simulink library "lib_all"  (Simulink 7.6)
settings: Solver=ode45  SolverMode=SingleTasking  StartTime=0.0  StopTime=10.0  RelTol=1e-3
values from neighbouring files (symbols this model uses):
  map_lib_03 = [6.8679 7.0159 7.4571 8.1849 9.1892 10.456 11.97 13.71 15.655 17.781 20.063 22.474 ... (803 elements, 73x11)]    [scr_simdata.mat]    (also in scr_simdata_all.mat)
  map_lib_06 = [2.5784 2.6727 2.9588 3.4453 4.1444 5.068 6.226 7.6242 9.2634 11.139 13.24 15.552 ... (803 elements, 73x11)]    [scr_simdata.mat]    (also in scr_simdata_all.mat)
  map_lib_09 = [0.0025784 0.0026727 0.0029588 0.0034453 0.0041444 0.005068 0.006226 0.0076242 0.0092634 0.011139 0.01324 0.015552 ... (803 elements, 73x11)]    [scr_simdata.mat]    (also in scr_simdata_all.mat)
  map_lib_10 = [2.74 2.75 2.76 2.77 2.78 2.79 2.8 2.81 2.82 2.83 2.84 2.85 ... (81 elements, 1x81)]    [scr_simdata.mat]    (also in scr_simdata_all.mat)
  map_lib_11 = [1.1983 1.2027 1.207 1.2113 1.2156 1.2198 1.2241 1.2283 1.2326 1.2368 1.241 1.2451 ... (81 elements, 1x81)]    [scr_simdata.mat]    (also in scr_simdata_all.mat)
  map_lib_12 = [2.74 2.75 2.76 2.77 2.78 2.79 2.8 2.81 2.82 2.83 2.84 2.85 ... (81 elements, 1x81)]    [scr_simdata.mat]    (also in scr_simdata_all.mat)
  map_lib_13 = [1.74 1.75 1.76 1.77 1.78 1.79 1.8 1.81 1.82 1.83 1.84 1.85 ... (81 elements, 1x81)]    [scr_simdata.mat]    (also in scr_simdata_all.mat)
  map_lib_16 = [0.00048828125 1.6159 1.8962 2.0821 2.2251 2.3426 2.4433 2.5318 2.611 2.683 2.749 2.8101 ... (1419 elements, 129x11)]    [scr_simdata.mat]    (also in scr_simdata_all.mat)
  map_mr2_01 = [0 800 1600 2400 3200 4000]    [scr_simdata.mat]    (also in scr_simdata_all.mat)
  map_mr2_02 = [1.32 1.32 1.33 1.34 1.36 1.38]    [scr_simdata.mat]    (also in scr_simdata_all.mat)
  map_mr2_03 = [0 800 1600 2400 3200 4000]    [scr_simdata.mat]    (also in scr_simdata_all.mat)
  map_mr2_04 = [1.32 1.32 1.33 1.34 1.36 1.38]    [scr_simdata.mat]    (also in scr_simdata_all.mat)
  map_mr2_05 = [0 800 1600 2400 3200 4000]    [scr_simdata.mat]    (also in scr_simdata_all.mat)
  map_mr2_06 = [1.32 1.32 1.33 1.34 1.36 1.38]    [scr_simdata.mat]    (also in scr_simdata_all.mat)
  Parameter1 = 6    [scr_simdata.mat]    (also in scr_simdata_all.mat)
  Parameter101 = 30    [scr_simdata.mat]    (also in scr_simdata_all.mat)
  Parameter102 = 12    [scr_simdata.mat]    (also in scr_simdata_all.mat)
  Parameter103 = -240    [scr_simdata.mat]    (also in scr_simdata_all.mat)
  Parameter108 = 48    [scr_simdata.mat]    (also in scr_simdata_all.mat)
  Parameter120 = 16    [scr_simdata.mat]    (also in scr_simdata_all.mat)
  Parameter121 = 87    [scr_simdata.mat]    (also in scr_simdata_all.mat)
  Parameter123 = 78    [scr_simdata.mat]    (also in scr_simdata_all.mat)
  Parameter124 = 60    [scr_simdata.mat]    (also in scr_simdata_all.mat)
  Parameter125 = -480    [scr_simdata.mat]    (also in scr_simdata_all.mat)
  Parameter130 = 96    [scr_simdata.mat]    (also in scr_simdata_all.mat)
  Parameter131 = 3    [scr_simdata.mat]    (also in scr_simdata_all.mat)
  Parameter144 = 3    [scr_simdata.mat]    (also in scr_simdata_all.mat)
  Parameter18 = 128    [scr_simdata.mat]    (also in scr_simdata_all.mat)
  Parameter19 = 16    [scr_simdata.mat]    (also in scr_simdata_all.mat)
  Parameter2 = 12    [scr_simdata.mat]    (also in scr_simdata_all.mat)
  Parameter20 = 7    [scr_simdata.mat]    (also in scr_simdata_all.mat)
  Parameter21 = 4    [scr_simdata.mat]    (also in scr_simdata_all.mat)
  Parameter3 = 18    [scr_simdata.mat]    (also in scr_simdata_all.mat)
  Parameter32 = 1    [scr_simdata.mat]    (also in scr_simdata_all.mat)
  Parameter34 = 24    [scr_simdata.mat]    (also in scr_simdata_all.mat)
  Parameter4 = 138    [scr_simdata.mat]    (also in scr_simdata_all.mat)
  Parameter42 = 48    [scr_simdata.mat]    (also in scr_simdata_all.mat)
  Parameter5 = 135    [scr_simdata.mat]    (also in scr_simdata_all.mat)
  Parameter55 = 0    [scr_simdata.mat]    (also in scr_simdata_all.mat)
  Parameter56 = 0    [scr_simdata.mat]    (also in scr_simdata_all.mat)
  Parameter57 = 1    [scr_simdata.mat]    (also in scr_simdata_all.mat)
  Parameter58 = 1    [scr_simdata.mat]    (also in scr_simdata_all.mat)
  Parameter59 = 2    [scr_simdata.mat]    (also in scr_simdata_all.mat)
  Parameter6 = 132    [scr_simdata.mat]    (also in scr_simdata_all.mat)
  Parameter60 = 2    [scr_simdata.mat]    (also in scr_simdata_all.mat)
  Parameter63 = 144    [scr_simdata.mat]    (also in scr_simdata_all.mat)
  Parameter7 = 126    [scr_simdata.mat]    (also in scr_simdata_all.mat)
  Parameter76 = 64    [scr_simdata.mat]    (also in scr_simdata_all.mat)
  Parameter77 = 135    [scr_simdata.mat]    (also in scr_simdata_all.mat)
  Parameter79 = 126    [scr_simdata.mat]    (also in scr_simdata_all.mat)
  Parameter80 = 108    [scr_simdata.mat]    (also in scr_simdata_all.mat)
  Parameter81 = 0    [scr_simdata.mat]    (also in scr_simdata_all.mat)
  Parameter86 = 0    [scr_simdata.mat]    (also in scr_simdata_all.mat)
  Parameter98 = 112    [scr_simdata.mat]    (also in scr_simdata_all.mat)
  Parameter99 = 39    [scr_simdata.mat]    (also in scr_simdata_all.mat)
undefined symbols (not defined in the file or in its folder): cylvolkp_hih_map2h, cylvolkp_map2h, pk1k_map2h
libraries: simulink x2
(part 6 of 36)

== system lib_all/SubSystem1023/SubSystem1189/SubSystem1194 ==
  note: ***編集禁止***
  element : Inport
  Inside_DSR : DataStoreRead  DataStoreName=DSM97
  Selector : Selector  IndexOptions=Index vector (port)  Indices=[1 3]  OutputSizes=1
  Output : Outport
  wires:
  Selector/1 -> Output/1
  element/1 -> Selector/2
  Inside_DSR/1 -> Selector/1

== system lib_all/SubSystem1023/SubSystem1189/SubSystem1195 ==
  note: ***編集禁止***
  element : Inport
  Inside_DSR : DataStoreRead  DataStoreName=DSM9
  Selector : Selector  IndexOptions=Index vector (port)  Indices=[1 3]  OutputSizes=1
  Output : Outport
  wires:
  Selector/1 -> Output/1
  element/1 -> Selector/2
  Inside_DSR/1 -> Selector/1

== system lib_all/SubSystem1196/SubSystem1200/SubSystem1201 ==
  note: ***編集禁止***
  In1 : Inport
  In2 : Inport  Port=2
  RO1 : RelationalOperator  Operator===  InputSameDT=on
  single1 : DataTypeConversion  OutDataTypeStr=single  SaturateOnIntegerOverflow=on  RndMeth=Zero
  single2 : DataTypeConversion  OutDataTypeStr=single  SaturateOnIntegerOverflow=on  RndMeth=Zero
  Out1 : Outport
  wires:
  RO1/1 -> Out1/1
  In2/1 -> single2/1
  In1/1 -> single1/1
  single1/1 -> RO1/1
  single2/1 -> RO1/2

== system lib_all/SubSystem1196/SubSystem1200/SubSystem1202 ==
  note: ***編集禁止***
  In1 : Inport
  In2 : Inport  Port=2
  RO1 : RelationalOperator  Operator===  InputSameDT=on
  single1 : DataTypeConversion  OutDataTypeStr=single  SaturateOnIntegerOverflow=on  RndMeth=Zero
  single2 : DataTypeConversion  OutDataTypeStr=single  SaturateOnIntegerOverflow=on  RndMeth=Zero
  Out1 : Outport
  wires:
  RO1/1 -> Out1/1
  In2/1 -> single2/1
  In1/1 -> single1/1
  single1/1 -> RO1/1
  single2/1 -> RO1/2

== system lib_all/SubSystem1196/SubSystem1200/SubSystem1203 ==
  note: ***編集禁止***
  In1 : Inport
  In2 : Inport  Port=2
  RO1 : RelationalOperator  Operator===  InputSameDT=on
  single1 : DataTypeConversion  OutDataTypeStr=single  SaturateOnIntegerOverflow=on  RndMeth=Zero
  single2 : DataTypeConversion  OutDataTypeStr=single  SaturateOnIntegerOverflow=on  RndMeth=Zero
  Out1 : Outport
  wires:
  RO1/1 -> Out1/1
  In2/1 -> single2/1
  In1/1 -> single1/1
  single1/1 -> RO1/1
  single2/1 -> RO1/2

== system lib_all/SubSystem1196/SubSystem1200/SubSystem1204 ==
  note: ***編集禁止***
  element : Inport
  Inside_DSR : DataStoreRead  DataStoreName=DSM97
  Selector : Selector  IndexOptions=Index vector (port)  Indices=[1 3]  OutputSizes=1
  Output : Outport
  wires:
  Selector/1 -> Output/1
  element/1 -> Selector/2
  Inside_DSR/1 -> Selector/1

== system lib_all/SubSystem1196/SubSystem1200/SubSystem1205 ==
  note: ***編集禁止***
  element : Inport
  Inside_DSR : DataStoreRead  DataStoreName=DSM9
  Selector : Selector  IndexOptions=Index vector (port)  Indices=[1 3]  OutputSizes=1
  Output : Outport
  wires:
  Selector/1 -> Output/1
  element/1 -> Selector/2
  Inside_DSR/1 -> Selector/1

== system lib_all/SubSystem1196/SubSystem1206/SubSystem1208 ==
  note: Text242
  Block3277 : Inport
  Block3278 : Inport  Port=2
  Block3279 : Inport  Port=3
  Block3280 : Inport  Port=4
  Block3281 : Constant  Value=0  OutDataTypeStr=double  SampleTime=-1
  Block3282 : Constant  OutDataTypeStr=double  SampleTime=-1
  Block3283 : Constant  Value=0  OutDataTypeStr=double  SampleTime=-1
  Block3284 : Gain  Gain=1/5  SaturateOnIntegerOverflow=on  OutDataTypeStr=Inherit: Same as input  ParamDataTypeStr=Inherit: Same as input
      settings: AttributesFormatString=Attribute652
  Block3285 : Product  OutDataTypeStr=double  SaturateOnIntegerOverflow=on  RndMeth=Zero  InputSameDT=on
  Block3286 : Sum  Inputs=-+  OutDataTypeStr=double  RndMeth=Zero  SaturateOnIntegerOverflow=on  InputSameDT=on
  Block3287 : Sum  OutDataTypeStr=double  RndMeth=Zero  SaturateOnIntegerOverflow=on  InputSameDT=on
  Block3288 : Sum  OutDataTypeStr=double  RndMeth=Zero  SaturateOnIntegerOverflow=on  InputSameDT=on
  Block3289 : Switch  Threshold=0.5  RndMeth=Zero  SaturateOnIntegerOverflow=on  InputSameDT=on
  SubSystem1210 : Subsystem
  SubSystem1211 : Subsystem
  SubSystem1217 : Subsystem
  Block3398 : Outport
  wires:
  Block3288/1 -> SubSystem1217/3(Block3319), SubSystem1211/2(Block3291)
  Block3277/1 -> Block3288/1
  Block3284/1 -> Block3287/2
  Block3285/1 -> Block3284/1
  Block3282/1 -> Block3288/2
  Block3281/1 -> Block3289/1
  Block3289/1 -> Block3398/1
  SubSystem1210/1(Out1) -> Block3289/2
  Block3283/1 -> SubSystem1210/2(In2)
  Block3279/1 -> SubSystem1211/3(Block3292)
  Block3287/1 -> SubSystem1210/1(In1), Block3289/3
  SubSystem1211/2(Block3316) -> Block3285/2
  Block3286/1 -> Block3285/1  "SigLabel110"
  SubSystem1217/2(Block3397) -> Block3286/2
  SubSystem1217/1(Block3396) -> Block3286/1, Block3287/1
  Block3280/1 -> SubSystem1217/2(Block3318)
  SubSystem1211/1(Block3315) -> SubSystem1217/1(Block3317)
  Block3278/1 -> SubSystem1211/1(Block3290)

== system lib_all/SubSystem1196/SubSystem1206/SubSystem1261 ==
  note: Text245
  Block3399 : Inport
  Block3400 : Inport  Port=2
  Block3401 : Inport  Port=3
  Block3402 : Inport  Port=4
  SubSystem1263 : Subsystem
  SubSystem1271 : Subsystem
  Block3444 : Outport
  wires:
  Block3401/1 -> SubSystem1263/3(Block3405)
  SubSystem1271/1(Block3443) -> Block3444/1
  Block3402/1 -> SubSystem1271/1(Block3436)
  SubSystem1263/1(Block3435) -> SubSystem1271/2(Block3437)
  Block3399/1 -> SubSystem1263/2(Block3404)
  Block3400/1 -> SubSystem1263/1(Block3403)

== system lib_all/SubSystem1196/SubSystem1273/SubSystem1275 ==
  note: Text254
  Block3452 : Inport
  Block3453 : Inport  Port=2
  Block3454 : Inport  Port=3
  Block3455 : Inport  Port=4
  Block3456 : Constant  Value=0  OutDataTypeStr=double  SampleTime=-1
  Block3457 : Constant  OutDataTypeStr=double  SampleTime=-1
  Block3458 : Constant  Value=0  OutDataTypeStr=double  SampleTime=-1
  Block3459 : Gain  Gain=1/5  SaturateOnIntegerOverflow=on  OutDataTypeStr=Inherit: Same as input  ParamDataTypeStr=Inherit: Same as input
      settings: AttributesFormatString=Attribute685
  Block3460 : Product  OutDataTypeStr=double  SaturateOnIntegerOverflow=on  RndMeth=Zero  InputSameDT=on
  Block3461 : Sum  Inputs=-+  OutDataTypeStr=double  RndMeth=Zero  SaturateOnIntegerOverflow=on  InputSameDT=on
  Block3462 : Sum  OutDataTypeStr=double  RndMeth=Zero  SaturateOnIntegerOverflow=on  InputSameDT=on
  Block3463 : Sum  OutDataTypeStr=double  RndMeth=Zero  SaturateOnIntegerOverflow=on  InputSameDT=on
  Block3464 : Switch  Threshold=0.5  RndMeth=Zero  SaturateOnIntegerOverflow=on  InputSameDT=on
  SubSystem1277 : Subsystem
  SubSystem1278 : Subsystem
  SubSystem1284 : Subsystem
  Block3573 : Outport
  wires:
  Block3463/1 -> SubSystem1284/3(Block3494), SubSystem1278/2(Block3466)
  Block3452/1 -> Block3463/1
  Block3459/1 -> Block3462/2
  Block3460/1 -> Block3459/1
  Block3457/1 -> Block3463/2
  Block3456/1 -> Block3464/1
  Block3464/1 -> Block3573/1
  SubSystem1277/1(Out1) -> Block3464/2
  Block3458/1 -> SubSystem1277/2(In2)
  Block3454/1 -> SubSystem1278/3(Block3467)
  Block3462/1 -> SubSystem1277/1(In1), Block3464/3
  SubSystem1278/2(Block3491) -> Block3460/2
  Block3461/1 -> Block3460/1  "SigLabel116"
  SubSystem1284/2(Block3572) -> Block3461/2
  SubSystem1284/1(Block3571) -> Block3461/1, Block3462/1
  Block3455/1 -> SubSystem1284/2(Block3493)
  SubSystem1278/1(Block3490) -> SubSystem1284/1(Block3492)
  Block3453/1 -> SubSystem1278/1(Block3465)

== system lib_all/SubSystem1196/SubSystem1273/SubSystem1328 ==
  note: Text257
  Block3574 : Inport
  Block3575 : Inport  Port=2
  Block3576 : Inport  Port=3
  Block3577 : Inport  Port=4
  SubSystem1330 : Subsystem
  SubSystem1338 : Subsystem
  Block3619 : Outport
  wires:
  Block3576/1 -> SubSystem1330/3(Block3580)
  SubSystem1338/1(Block3618) -> Block3619/1
  Block3577/1 -> SubSystem1338/1(Block3611)
  SubSystem1330/1(Block3610) -> SubSystem1338/2(Block3612)
  Block3574/1 -> SubSystem1330/2(Block3579)
  Block3575/1 -> SubSystem1330/1(Block3578)

== system lib_all/SubSystem1196/SubSystem1340/SubSystem1342 ==
  note: Text261
  Block3625 : Inport
  Block3626 : Inport  Port=2
  Block3627 : Inport  Port=3
  Block3628 : Constant  Value=0  OutDataTypeStr=double  SampleTime=-1
  Block3629 : Constant  Value=720  OutDataTypeStr=double  SampleTime=-1
  Block3630 : Gain  Gain=5  SaturateOnIntegerOverflow=on  OutDataTypeStr=Inherit: Same as input  ParamDataTypeStr=Inherit: Same as input
      settings: AttributesFormatString=Attribute716
  Block3631 : Sum  Inputs=+-  OutDataTypeStr=double  RndMeth=Zero  SaturateOnIntegerOverflow=on  InputSameDT=on
  SubSystem1343 : Subsystem
      settings: RTWFcnNameOpts=Use subsystem name
  SubSystem1348 : Subsystem
      settings: RTWSystemCode=Inline
  Block3657 : Outport
  wires:
  SubSystem1348/1(Block3656) -> Block3657/1
  Block3628/1 -> SubSystem1348/3(Block3653)
  Block3629/1 -> SubSystem1348/1(Block3651)
  Block3631/1 -> SubSystem1348/2(Block3652)
  Block3627/1 -> Block3631/2
  Block3626/1 -> SubSystem1343/2(Block3633)
  Block3625/1 -> SubSystem1343/1(Block3632)
  SubSystem1343/1(Block3650) -> Block3630/1
  Block3630/1 -> Block3631/1

== system lib_all/SubSystem1196/SubSystem1340/SubSystem1350 ==
  note: Text262
  Block3658 : Inport
  Block3659 : Inport  Port=2
  Block3660 : Constant  Value=360  OutDataTypeStr=double  SampleTime=-1
  Block3661 : Constant  Value=360  OutDataTypeStr=double  SampleTime=-1
  Block3662 : Sum  Inputs=+-  OutDataTypeStr=double  RndMeth=Zero  SaturateOnIntegerOverflow=on  InputSameDT=on
  Block3663 : Switch  Threshold=0.5  RndMeth=Zero  SaturateOnIntegerOverflow=on  InputSameDT=on
  Block3664 : Lookup2D  RowIndex=map_lib_08  ColumnIndex=map_lib_07  Table=map_lib_09  SaturateOnIntegerOverflow=on  InputSameDT=on
      settings: AttributesFormatString=<%<OutputValues>>\nAttribute725
  SubSystem1351 : Subsystem
  Block3665 : Outport
  wires:
  Block3664/1 -> Block3665/1
  Block3660/1 -> Block3662/2
  Block3662/1 -> Block3663/1
  SubSystem1351/1(Out1) -> Block3663/2
  Block3661/1 -> SubSystem1351/2(In2)
  Block3659/1 -> Block3662/1, Block3663/3, SubSystem1351/1(In1)
  Block3658/1 -> Block3664/2
  Block3663/1 -> Block3664/1  "SigLabel120"

== system lib_all/SubSystem1196/SubSystem1352/SubSystem1354 ==
  note: Text264
  Block3671 : Inport
  Block3672 : Inport  Port=2
  Block3673 : Inport  Port=3
  Block3674 : Constant  Value=0  OutDataTypeStr=double  SampleTime=-1
  Block3675 : Constant  Value=720  OutDataTypeStr=double  SampleTime=-1
  Block3676 : Gain  Gain=5  SaturateOnIntegerOverflow=on  OutDataTypeStr=Inherit: Same as input  ParamDataTypeStr=Inherit: Same as input
      settings: AttributesFormatString=Attribute729
  Block3677 : Sum  Inputs=+-  OutDataTypeStr=double  RndMeth=Zero  SaturateOnIntegerOverflow=on  InputSameDT=on
  SubSystem1355 : Subsystem
      settings: RTWFcnNameOpts=Use subsystem name
  SubSystem1360 : Subsystem
      settings: RTWSystemCode=Inline
  Block3703 : Outport
  wires:
  SubSystem1360/1(Block3702) -> Block3703/1
  Block3674/1 -> SubSystem1360/3(Block3699)
  Block3675/1 -> SubSystem1360/1(Block3697)
  Block3677/1 -> SubSystem1360/2(Block3698)
  Block3673/1 -> Block3677/2
  Block3672/1 -> SubSystem1355/2(Block3679)
  Block3671/1 -> SubSystem1355/1(Block3678)
  SubSystem1355/1(Block3696) -> Block3676/1
  Block3676/1 -> Block3677/1

== system lib_all/SubSystem1196/SubSystem1352/SubSystem1362 ==
  note: Text265
  Block3704 : Inport
  Block3705 : Inport  Port=2
  Block3706 : Constant  Value=360  OutDataTypeStr=double  SampleTime=-1
  Block3707 : Constant  Value=360  OutDataTypeStr=double  SampleTime=-1
  Block3708 : Sum  Inputs=+-  OutDataTypeStr=double  RndMeth=Zero  SaturateOnIntegerOverflow=on  InputSameDT=on
  Block3709 : Switch  Threshold=0.5  RndMeth=Zero  SaturateOnIntegerOverflow=on  InputSameDT=on
  Block3710 : Lookup2D  RowIndex=map_lib_08  ColumnIndex=map_lib_07  Table=map_lib_09  SaturateOnIntegerOverflow=on  InputSameDT=on
      settings: AttributesFormatString=<%<OutputValues>>\nAttribute738
  SubSystem1363 : Subsystem
  Block3711 : Outport
  wires:
  Block3710/1 -> Block3711/1
  Block3706/1 -> Block3708/2
  Block3708/1 -> Block3709/1
  SubSystem1363/1(Out1) -> Block3709/2
  Block3707/1 -> SubSystem1363/2(In2)
  Block3705/1 -> Block3708/1, Block3709/3, SubSystem1363/1(In1)
  Block3704/1 -> Block3710/2
  Block3709/1 -> Block3710/1  "SigLabel121"

== system lib_all/SubSystem1364/SubSystem1366/SubSystem1368 ==
  note: ***編集禁止***
  In1 : Inport
  In2 : Inport  Port=2
  LO1 : Logic
  RO1 : RelationalOperator  Operator=<  InputSameDT=on
  RO2 : RelationalOperator  Operator=~=  InputSameDT=on
  single1 : DataTypeConversion  OutDataTypeStr=single  SaturateOnIntegerOverflow=on  RndMeth=Zero
  single2 : DataTypeConversion  OutDataTypeStr=single  SaturateOnIntegerOverflow=on  RndMeth=Zero
  Out1 : Outport
  wires:
  LO1/1 -> Out1/1
  RO2/1 -> LO1/2
  RO1/1 -> LO1/1
  single2/1 -> RO2/2, RO1/2
  single1/1 -> RO2/1, RO1/1
  In1/1 -> single1/1
  In2/1 -> single2/1

== system lib_all/SubSystem1364/SubSystem1366/SubSystem1369 ==
  note: Text270
  note: Text269
  Block3744 : Inport
  Block3745 : Inport  Port=2
  Block3746 : Inport  Port=3
  Block3747 : Constant  Value=720  OutDataTypeStr=double  SampleTime=-1
  Block3748 : Constant  Value=0  OutDataTypeStr=double  SampleTime=-1
  Block3749 : Constant  Value=0  OutDataTypeStr=double  SampleTime=-1
  Block3750 : Constant  Value=5  OutDataTypeStr=double  SampleTime=-1
  Block3751 : DataStoreRead  DataStoreName=DSM50
  Block3752 : DataStoreRead  DataStoreName=DSM35
  Block3753 : Gain  Gain=5  SaturateOnIntegerOverflow=on  OutDataTypeStr=Inherit: Same as input  ParamDataTypeStr=Inherit: Same as input
      settings: AttributesFormatString=Attribute752
  Block3754 : Gain  Gain=5  SaturateOnIntegerOverflow=on  OutDataTypeStr=Inherit: Same as input  ParamDataTypeStr=Inherit: Same as input
      settings: AttributesFormatString=Attribute753
  Block3755 : Gain  Gain=1/5  SaturateOnIntegerOverflow=on  OutDataTypeStr=Inherit: Same as input  ParamDataTypeStr=Inherit: Same as input
      settings: AttributesFormatString=Attribute754
  Block3756 : Product  Inputs=*/  OutDataTypeStr=double  SaturateOnIntegerOverflow=on  RndMeth=Zero  InputSameDT=on
  Block3757 : Rounding
  Block3758 : Rounding
  Block3759 : Sum  Inputs=+-+-  OutDataTypeStr=double  RndMeth=Zero  SaturateOnIntegerOverflow=on  InputSameDT=on
  Block3760 : Sum  Inputs=-+  OutDataTypeStr=double  RndMeth=Zero  SaturateOnIntegerOverflow=on  InputSameDT=on
  SubSystem1370 : Subsystem  TreatAsAtomicUnit=on  mask(***ATC.DIVISION***){bd_version=1.01.A, r0=チェック有り, r1=, r2=, r3=, r4=, r5=, r6=, r7=, r8=, r9=, c0=, c1=, c2=, c3=, c4=, c5=, c6=, c7=, c8=, c9=}
      mask prompts: bd_version "Version"
      mask help: これはAutoCからのブロックです．編集禁止．
      settings: OpenFcn=warndlg('変更禁止!');
      mask Help: web(ui_blockhelpmng(gcb));
  SubSystem1371 : Subsystem  TreatAsAtomicUnit=on  mask(***ATC.DSRVEC***){bd_version=1.01.A, r0=DSM95, r1=-1, r2=, r3=, r4=, r5=, r6=, r7=, r8=, r9=, c0=, c1=, c2=, c3=, c4=, c5=, c6=, c7=, c8=, c9=}
      mask prompts: bd_version "Version"
      mask help: これはAutoCからのブロックです．編集禁止．
      settings: OpenFcn=warndlg('変更禁止!');
      mask Help: web(ui_blockhelpmng(gcb));
      mask Display: disp('ATC.DSRVEC')
  SubSystem1372 : Subsystem
      settings: RTWSystemCode=Inline
  Block3769 : Outport
  Block3770 : Outport  Port=2
  wires:
  Block3752/1 -> SubSystem1370/2(div)
  Block3760/1 -> Block3770/1
  Block3753/1 -> Block3760/1
  Block3757/1 -> Block3753/1
  Block3756/1 -> Block3757/1
  Block3750/1 -> Block3756/2
  Block3745/1 -> SubSystem1371/1(element)
  Block3749/1 -> SubSystem1372/3(Block3763)
  Block3759/1 -> SubSystem1372/2(Block3762)  "SigLabel135"
  Block3747/1 -> SubSystem1372/1(Block3761)
  Block3746/1 -> Block3759/4
  SubSystem1372/1(Block3768) -> Block3756/1, Block3760/2, Block3755/1
  Block3744/1 -> Block3754/1
  SubSystem1370/1(Out) -> Block3759/3  "SigLabel134"
  Block3748/1 -> SubSystem1370/3(init)
  SubSystem1371/1(Output) -> SubSystem1370/1(mult)
  Block3751/1 -> Block3759/2
  Block3754/1 -> Block3759/1
  Block3755/1 -> Block3758/1
  Block3758/1 -> Block3769/1

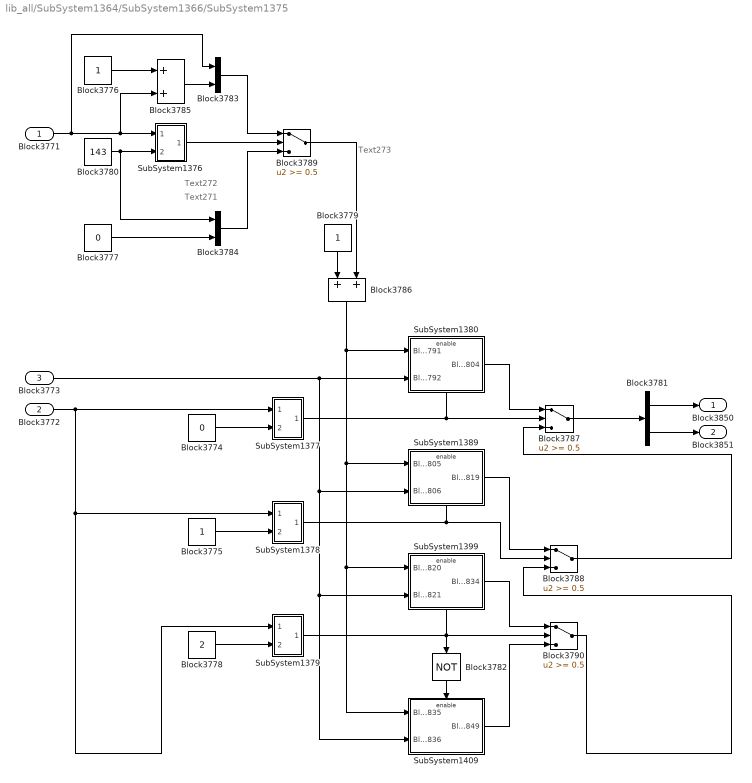
== system lib_all/SubSystem1364/SubSystem1366/SubSystem1375 ==
  note: Text273
  note: Text272
  note: Text271
  Block3771 : Inport
  Block3772 : Inport  Port=2
  Block3773 : Inport  Port=3
  Block3774 : Constant  Value=0  OutDataTypeStr=double  SampleTime=-1
  Block3775 : Constant  OutDataTypeStr=double  SampleTime=-1
  Block3776 : Constant  OutDataTypeStr=double  SampleTime=-1
  Block3777 : Constant  Value=0  OutDataTypeStr=double  SampleTime=-1
  Block3778 : Constant  Value=2  OutDataTypeStr=double  SampleTime=-1
  Block3779 : Constant  OutDataTypeStr=double  SampleTime=-1
  Block3780 : Constant  Value=143  OutDataTypeStr=double  SampleTime=-1
  Block3781 : Demux  Outputs=2
  Block3782 : Logic  Operator=NOT
  Block3783 : Mux  Inputs=2
  Block3784 : Mux  Inputs=2
  Block3785 : Sum  OutDataTypeStr=double  RndMeth=Zero  SaturateOnIntegerOverflow=on  InputSameDT=on
  Block3786 : Sum  OutDataTypeStr=double  RndMeth=Zero  SaturateOnIntegerOverflow=on  InputSameDT=on
  Block{3787..3790} (4 alike) : Switch  Threshold=0.5  RndMeth=Zero  SaturateOnIntegerOverflow=on  InputSameDT=on
  SubSystem1376 : Subsystem
  SubSystem1377 : Subsystem
  SubSystem1378 : Subsystem
  SubSystem1379 : Subsystem
  SubSystem1380 : Subsystem  TreatAsAtomicUnit=on
  SubSystem1389 : Subsystem  TreatAsAtomicUnit=on
  SubSystem1399 : Subsystem  TreatAsAtomicUnit=on
  SubSystem1409 : Subsystem  TreatAsAtomicUnit=on
  Block3850 : Outport
  Block3851 : Outport  Port=2
  wires:
  Block3773/1 -> SubSystem1409/2(Block3836), SubSystem1399/2(Block3821), SubSystem1389/2(Block3806), SubSystem1380/2(Block3792)
  Block3790/1 -> Block3788/3
  SubSystem1409/1(Block3849) -> Block3790/3
  SubSystem1399/1(Block3834) -> Block3790/1
  Block3782/1 -> SubSystem1409/Enable
  SubSystem1379/1(Out1) -> Block3790/2, SubSystem1399/Enable, Block3782/1
  Block3778/1 -> SubSystem1379/2(In2)
  Block3783/1 -> Block3789/1
  Block3785/1 -> Block3783/2
  Block3784/1 -> Block3789/3
  Block3777/1 -> Block3784/2
  Block3776/1 -> Block3785/1
  SubSystem1376/1(Out1) -> Block3789/2
  Block3787/1 -> Block3781/1
  Block3781/2 -> Block3851/1
  Block3781/1 -> Block3850/1
  Block3786/1 -> SubSystem1409/1(Block3835), SubSystem1399/1(Block3820), SubSystem1389/1(Block3805), SubSystem1380/1(Block3791)
  Block3788/1 -> Block3787/3
  SubSystem1389/1(Block3819) -> Block3788/1
  SubSystem1378/1(Out1) -> SubSystem1389/Enable, Block3788/2
  Block3775/1 -> SubSystem1378/2(In2)
  SubSystem1380/1(Block3804) -> Block3787/1
  SubSystem1377/1(Out1) -> SubSystem1380/Enable, Block3787/2
  Block3774/1 -> SubSystem1377/2(In2)
  Block3772/1 -> SubSystem1379/1(In1), SubSystem1378/1(In1), SubSystem1377/1(In1)
  Block3780/1 -> Block3784/1, SubSystem1376/2(In2)
  Block3771/1 -> Block3783/1, Block3785/2, SubSystem1376/1(In1)
  Block3779/1 -> Block3786/1
  Block3789/1 -> Block3786/2

== system lib_all/SubSystem1364/SubSystem1419/SubSystem1421 ==
  note: ***編集禁止***
  In1 : Inport
  In2 : Inport  Port=2
  LO1 : Logic
  RO1 : RelationalOperator  Operator=<  InputSameDT=on
  RO2 : RelationalOperator  Operator=~=  InputSameDT=on
  single1 : DataTypeConversion  OutDataTypeStr=single  SaturateOnIntegerOverflow=on  RndMeth=Zero
  single2 : DataTypeConversion  OutDataTypeStr=single  SaturateOnIntegerOverflow=on  RndMeth=Zero
  Out1 : Outport
  wires:
  LO1/1 -> Out1/1
  RO2/1 -> LO1/2
  RO1/1 -> LO1/1
  single2/1 -> RO2/2, RO1/2
  single1/1 -> RO2/1, RO1/1
  In1/1 -> single1/1
  In2/1 -> single2/1

== system lib_all/SubSystem1364/SubSystem1419/SubSystem1422 ==
  note: Text276
  note: Text275
  Block3866 : Inport
  Block3867 : Inport  Port=2
  Block3868 : Inport  Port=3
  Block3869 : Constant  Value=720  OutDataTypeStr=double  SampleTime=-1
  Block3870 : Constant  Value=0  OutDataTypeStr=double  SampleTime=-1
  Block3871 : Constant  Value=0  OutDataTypeStr=double  SampleTime=-1
  Block3872 : Constant  Value=5  OutDataTypeStr=double  SampleTime=-1
  Block3873 : DataStoreRead  DataStoreName=DSM50
  Block3874 : DataStoreRead  DataStoreName=DSM35
  Block3875 : Gain  Gain=5  SaturateOnIntegerOverflow=on  OutDataTypeStr=Inherit: Same as input  ParamDataTypeStr=Inherit: Same as input
      settings: AttributesFormatString=Attribute771
  Block3876 : Gain  Gain=5  SaturateOnIntegerOverflow=on  OutDataTypeStr=Inherit: Same as input  ParamDataTypeStr=Inherit: Same as input
      settings: AttributesFormatString=Attribute772
  Block3877 : Gain  Gain=1/5  SaturateOnIntegerOverflow=on  OutDataTypeStr=Inherit: Same as input  ParamDataTypeStr=Inherit: Same as input
      settings: AttributesFormatString=Attribute773
  Block3878 : Product  Inputs=*/  OutDataTypeStr=double  SaturateOnIntegerOverflow=on  RndMeth=Zero  InputSameDT=on
  Block3879 : Rounding
  Block3880 : Rounding
  Block3881 : Sum  Inputs=+-+-  OutDataTypeStr=double  RndMeth=Zero  SaturateOnIntegerOverflow=on  InputSameDT=on
  Block3882 : Sum  Inputs=-+  OutDataTypeStr=double  RndMeth=Zero  SaturateOnIntegerOverflow=on  InputSameDT=on
  SubSystem1423 : Subsystem  TreatAsAtomicUnit=on  mask(***ATC.DIVISION***){bd_version=1.01.A, r0=チェック有り, r1=, r2=, r3=, r4=, r5=, r6=, r7=, r8=, r9=, c0=, c1=, c2=, c3=, c4=, c5=, c6=, c7=, c8=, c9=}
      mask prompts: bd_version "Version"
      mask help: これはAutoCからのブロックです．編集禁止．
      settings: OpenFcn=warndlg('変更禁止!');
      mask Help: web(ui_blockhelpmng(gcb));
  SubSystem1424 : Subsystem  TreatAsAtomicUnit=on  mask(***ATC.DSRVEC***){bd_version=1.01.A, r0=DSM95, r1=-1, r2=, r3=, r4=, r5=, r6=, r7=, r8=, r9=, c0=, c1=, c2=, c3=, c4=, c5=, c6=, c7=, c8=, c9=}
      mask prompts: bd_version "Version"
      mask help: これはAutoCからのブロックです．編集禁止．
      settings: OpenFcn=warndlg('変更禁止!');
      mask Help: web(ui_blockhelpmng(gcb));
      mask Display: disp('ATC.DSRVEC')
  SubSystem1425 : Subsystem
      settings: RTWSystemCode=Inline
  Block3891 : Outport
  Block3892 : Outport  Port=2
  wires:
  Block3874/1 -> SubSystem1423/2(div)
  Block3882/1 -> Block3892/1
  Block3875/1 -> Block3882/1
  Block3879/1 -> Block3875/1
  Block3878/1 -> Block3879/1
  Block3872/1 -> Block3878/2
  Block3867/1 -> SubSystem1424/1(element)
  Block3871/1 -> SubSystem1425/3(Block3885)
  Block3881/1 -> SubSystem1425/2(Block3884)  "SigLabel138"
  Block3869/1 -> SubSystem1425/1(Block3883)
  Block3868/1 -> Block3881/4
  SubSystem1425/1(Block3890) -> Block3878/1, Block3882/2, Block3877/1
  Block3866/1 -> Block3876/1
  SubSystem1423/1(Out) -> Block3881/3  "SigLabel137"
  Block3870/1 -> SubSystem1423/3(init)
  SubSystem1424/1(Output) -> SubSystem1423/1(mult)
  Block3873/1 -> Block3881/2
  Block3876/1 -> Block3881/1
  Block3877/1 -> Block3880/1
  Block3880/1 -> Block3891/1

== system lib_all/SubSystem1364/SubSystem1419/SubSystem1428 ==
  note: Text279
  note: Text278
  note: Text277
  Block3893 : Inport
  Block3894 : Inport  Port=2
  Block3895 : Inport  Port=3
  Block3896 : Constant  Value=0  OutDataTypeStr=double  SampleTime=-1
  Block3897 : Constant  OutDataTypeStr=double  SampleTime=-1
  Block3898 : Constant  OutDataTypeStr=double  SampleTime=-1
  Block3899 : Constant  Value=0  OutDataTypeStr=double  SampleTime=-1
  Block3900 : Constant  Value=2  OutDataTypeStr=double  SampleTime=-1
  Block3901 : Constant  OutDataTypeStr=double  SampleTime=-1
  Block3902 : Constant  Value=143  OutDataTypeStr=double  SampleTime=-1
  Block3903 : Demux  Outputs=2
  Block3904 : Logic  Operator=NOT
  Block3905 : Mux  Inputs=2
  Block3906 : Mux  Inputs=2
  Block3907 : Sum  OutDataTypeStr=double  RndMeth=Zero  SaturateOnIntegerOverflow=on  InputSameDT=on
  Block3908 : Sum  OutDataTypeStr=double  RndMeth=Zero  SaturateOnIntegerOverflow=on  InputSameDT=on
  Block{3909..3912} (4 alike) : Switch  Threshold=0.5  RndMeth=Zero  SaturateOnIntegerOverflow=on  InputSameDT=on
  SubSystem1429 : Subsystem
  SubSystem1430 : Subsystem
  SubSystem1431 : Subsystem
  SubSystem1432 : Subsystem
  SubSystem1433 : Subsystem  TreatAsAtomicUnit=on
  SubSystem1442 : Subsystem  TreatAsAtomicUnit=on
  SubSystem1452 : Subsystem  TreatAsAtomicUnit=on
  SubSystem1462 : Subsystem  TreatAsAtomicUnit=on
  Block3972 : Outport
  Block3973 : Outport  Port=2
  wires:
  Block3895/1 -> SubSystem1462/2(Block3958), SubSystem1452/2(Block3943), SubSystem1442/2(Block3928), SubSystem1433/2(Block3914)
  Block3912/1 -> Block3910/3
  SubSystem1462/1(Block3971) -> Block3912/3
  SubSystem1452/1(Block3956) -> Block3912/1
  Block3904/1 -> SubSystem1462/Enable
  SubSystem1432/1(Out1) -> Block3912/2, SubSystem1452/Enable, Block3904/1
  Block3900/1 -> SubSystem1432/2(In2)
  Block3905/1 -> Block3911/1
  Block3907/1 -> Block3905/2
  Block3906/1 -> Block3911/3
  Block3899/1 -> Block3906/2
  Block3898/1 -> Block3907/1
  SubSystem1429/1(Out1) -> Block3911/2
  Block3909/1 -> Block3903/1
  Block3903/2 -> Block3973/1
  Block3903/1 -> Block3972/1
  Block3908/1 -> SubSystem1462/1(Block3957), SubSystem1452/1(Block3942), SubSystem1442/1(Block3927), SubSystem1433/1(Block3913)
  Block3910/1 -> Block3909/3
  SubSystem1442/1(Block3941) -> Block3910/1
  SubSystem1431/1(Out1) -> SubSystem1442/Enable, Block3910/2
  Block3897/1 -> SubSystem1431/2(In2)
  SubSystem1433/1(Block3926) -> Block3909/1
  SubSystem1430/1(Out1) -> SubSystem1433/Enable, Block3909/2
  Block3896/1 -> SubSystem1430/2(In2)
  Block3894/1 -> SubSystem1432/1(In1), SubSystem1431/1(In1), SubSystem1430/1(In1)
  Block3902/1 -> Block3906/1, SubSystem1429/2(In2)
  Block3893/1 -> Block3905/1, Block3907/2, SubSystem1429/1(In1)
  Block3901/1 -> Block3908/1
  Block3911/1 -> Block3908/2

== system lib_all/SubSystem1364/SubSystem1472/SubSystem1473 ==
  note: ***編集禁止***
  In1 : Inport
  In2 : Inport  Port=2
  RO1 : RelationalOperator  Operator===  InputSameDT=on
  single1 : DataTypeConversion  OutDataTypeStr=single  SaturateOnIntegerOverflow=on  RndMeth=Zero
  single2 : DataTypeConversion  OutDataTypeStr=single  SaturateOnIntegerOverflow=on  RndMeth=Zero
  Out1 : Outport
  wires:
  RO1/1 -> Out1/1
  In2/1 -> single2/1
  In1/1 -> single1/1
  single1/1 -> RO1/1
  single2/1 -> RO1/2

== system lib_all/SubSystem1364/SubSystem1472/SubSystem1474 ==
  note: ***編集禁止***
  In1 : Inport
  In2 : Inport  Port=2
  RO1 : RelationalOperator  Operator===  InputSameDT=on
  single1 : DataTypeConversion  OutDataTypeStr=single  SaturateOnIntegerOverflow=on  RndMeth=Zero
  single2 : DataTypeConversion  OutDataTypeStr=single  SaturateOnIntegerOverflow=on  RndMeth=Zero
  Out1 : Outport
  wires:
  RO1/1 -> Out1/1
  In2/1 -> single2/1
  In1/1 -> single1/1
  single1/1 -> RO1/1
  single2/1 -> RO1/2

== system lib_all/SubSystem1364/SubSystem1472/SubSystem1475 ==
  note: ***編集禁止***
  In1 : Inport
  In2 : Inport  Port=2
  RO1 : RelationalOperator  Operator===  InputSameDT=on
  single1 : DataTypeConversion  OutDataTypeStr=single  SaturateOnIntegerOverflow=on  RndMeth=Zero
  single2 : DataTypeConversion  OutDataTypeStr=single  SaturateOnIntegerOverflow=on  RndMeth=Zero
  Out1 : Outport
  wires:
  RO1/1 -> Out1/1
  In2/1 -> single2/1
  In1/1 -> single1/1
  single1/1 -> RO1/1
  single2/1 -> RO1/2

== system lib_all/SubSystem1364/SubSystem1472/SubSystem1476 ==
  note: ***編集禁止***
  element : Inport
  Inside_DSR : DataStoreRead  DataStoreName=DSM97
  Selector : Selector  IndexOptions=Index vector (port)  Indices=[1 3]  OutputSizes=1
  Output : Outport
  wires:
  Selector/1 -> Output/1
  element/1 -> Selector/2
  Inside_DSR/1 -> Selector/1

== system lib_all/SubSystem1364/SubSystem1472/SubSystem1477 ==
  note: ***編集禁止***
  element : Inport
  Inside_DSR : DataStoreRead  DataStoreName=DSM9
  Selector : Selector  IndexOptions=Index vector (port)  Indices=[1 3]  OutputSizes=1
  Output : Outport
  wires:
  Selector/1 -> Output/1
  element/1 -> Selector/2
  Inside_DSR/1 -> Selector/1

== system lib_all/SubSystem1487/SubSystem1490/SubSystem1491 ==
  note: ***編集禁止***
  mult : Inport
  div : Inport  Port=2
  init : Inport  Port=3
  Product : Subsystem  TreatAsAtomicUnit=on
      settings: RTWSystemCode=Inline
  Out : Outport
  wires:
  Product/1(Out) -> Out/1
  mult/1 -> Product/1(mult)
  div/1 -> Product/2(div)
  init/1 -> Product/3(init)

== system lib_all/SubSystem1487/SubSystem1490/SubSystem1492 ==
  note: ***編集禁止***
  element : Inport
  Inside_DSR : DataStoreRead  DataStoreName=DSM95
  Selector : Selector  IndexOptions=Index vector (port)  Indices=[1 3]  OutputSizes=1
  Output : Outport
  wires:
  Selector/1 -> Output/1
  element/1 -> Selector/2
  Inside_DSR/1 -> Selector/1

== system lib_all/SubSystem1487/SubSystem1490/SubSystem1493 ==
  Block4050 : Inport
  Block4051 : Inport  Port=2
  Block4052 : Inport  Port=3
  Block4053 : Sum  OutDataTypeStr=double  RndMeth=Zero  SaturateOnIntegerOverflow=on  InputSameDT=on
  Block4054 : Sum  Inputs=+-  OutDataTypeStr=double  RndMeth=Zero  SaturateOnIntegerOverflow=on  InputSameDT=on
  Block4055 : Switch  Threshold=0.5  RndMeth=Zero  SaturateOnIntegerOverflow=on  InputSameDT=on
  Block4056 : Switch  Threshold=0.5  RndMeth=Zero  SaturateOnIntegerOverflow=on  InputSameDT=on
  SubSystem1494 : Subsystem
  SubSystem1495 : Subsystem
  Block4057 : Outport
  wires:
  Block4051/1 -> SubSystem1494/1(In1), Block4054/1, SubSystem1495/1(In1), Block4056/3, Block4053/2
  Block4050/1 -> Block4053/1, Block4054/2, SubSystem1494/2(In2)
  SubSystem1494/1(Out1) -> Block4055/2
  Block4052/1 -> SubSystem1495/2(In2)
  SubSystem1495/1(Out1) -> Block4056/2
  Block4056/1 -> Block4055/3
  Block4053/1 -> Block4056/1
  Block4055/1 -> Block4057/1
  Block4054/1 -> Block4055/1

== system lib_all/SubSystem1487/SubSystem1496/SubSystem1497 ==
  note: ***編集禁止***
  In1 : Inport
  In2 : Inport  Port=2
  LO1 : Logic
  RO1 : RelationalOperator  Operator=<  InputSameDT=on
  RO2 : RelationalOperator  Operator=~=  InputSameDT=on
  single1 : DataTypeConversion  OutDataTypeStr=single  SaturateOnIntegerOverflow=on  RndMeth=Zero
  single2 : DataTypeConversion  OutDataTypeStr=single  SaturateOnIntegerOverflow=on  RndMeth=Zero
  Out1 : Outport
  wires:
  LO1/1 -> Out1/1
  RO2/1 -> LO1/2
  RO1/1 -> LO1/1
  In2/1 -> single2/1
  In1/1 -> single1/1
  single1/1 -> RO2/1, RO1/1
  single2/1 -> RO2/2, RO1/2

== system lib_all/SubSystem1487/SubSystem1496/SubSystem1498 ==
  note: ***編集禁止***
  In1 : Inport
  In2 : Inport  Port=2
  RO1 : RelationalOperator  Operator===  InputSameDT=on
  single1 : DataTypeConversion  OutDataTypeStr=single  SaturateOnIntegerOverflow=on  RndMeth=Zero
  single2 : DataTypeConversion  OutDataTypeStr=single  SaturateOnIntegerOverflow=on  RndMeth=Zero
  Out1 : Outport
  wires:
  RO1/1 -> Out1/1
  In2/1 -> single2/1
  In1/1 -> single1/1
  single1/1 -> RO1/1
  single2/1 -> RO1/2

== system lib_all/SubSystem1487/SubSystem1496/SubSystem1499 ==
  note: ***編集禁止***
  In1 : Inport
  In2 : Inport  Port=2
  RO1 : RelationalOperator  Operator===  InputSameDT=on
  single1 : DataTypeConversion  OutDataTypeStr=single  SaturateOnIntegerOverflow=on  RndMeth=Zero
  single2 : DataTypeConversion  OutDataTypeStr=single  SaturateOnIntegerOverflow=on  RndMeth=Zero
  Out1 : Outport
  wires:
  RO1/1 -> Out1/1
  In2/1 -> single2/1
  In1/1 -> single1/1
  single1/1 -> RO1/1
  single2/1 -> RO1/2

== system lib_all/SubSystem1487/SubSystem1496/SubSystem1500 ==
  note: ***編集禁止***
  In1 : Inport
  In2 : Inport  Port=2
  RO1 : RelationalOperator  Operator===  InputSameDT=on
  single1 : DataTypeConversion  OutDataTypeStr=single  SaturateOnIntegerOverflow=on  RndMeth=Zero
  single2 : DataTypeConversion  OutDataTypeStr=single  SaturateOnIntegerOverflow=on  RndMeth=Zero
  Out1 : Outport
  wires:
  RO1/1 -> Out1/1
  In2/1 -> single2/1
  In1/1 -> single1/1
  single1/1 -> RO1/1
  single2/1 -> RO1/2

== system lib_all/SubSystem1487/SubSystem1496/SubSystem1501 ==
  Block4080 : Inport
  Block4081 : Inport  Port=2
  Block4082 : EnablePort
  Block4083 : Demux  Outputs=2
  Block4084 : Mux  Inputs=2
  SubSystem1502 : Subsystem
  SubSystem1506 : Subsystem
  Block4093 : Outport
  wires:
  Block4080/1 -> Block4083/1
  Block4081/1 -> SubSystem1506/1(Block4089), SubSystem1502/1(Block4085)
  Block4083/1 -> SubSystem1502/2(Block4086)
  Block4083/2 -> SubSystem1506/2(Block4090)
  SubSystem1502/1(Block4088) -> Block4084/1
  SubSystem1506/1(Block4092) -> Block4084/2
  Block4084/1 -> Block4093/1

== system lib_all/SubSystem1487/SubSystem1496/SubSystem1510 ==
  Block4094 : Inport
  Block4095 : Inport  Port=2
  Block4096 : EnablePort
  Block4097 : Demux  Outputs=2
  Block4098 : Mux  Inputs=2
  Block4099 : Sum  OutDataTypeStr=double  RndMeth=Zero  SaturateOnIntegerOverflow=on  InputSameDT=on
  SubSystem1511 : Subsystem  TreatAsAtomicUnit=on  mask(***ATC.DSRVEC***){bd_version=1.01.A, r0=DSM1, r1=-1, r2=, r3=, r4=, r5=, r6=, r7=, r8=, r9=, c0=, c1=, c2=, c3=, c4=, c5=, c6=, c7=, c8=, c9=}
      mask prompts: bd_version "Version"
      mask help: これはAutoCからのブロックです．編集禁止．
      settings: OpenFcn=warndlg('変更禁止!');
      mask Help: web(ui_blockhelpmng(gcb));
      mask Display: disp('ATC.DSRVEC')
  SubSystem1512 : Subsystem
  SubSystem1516 : Subsystem
  Block4108 : Outport
  wires:
  Block4094/1 -> Block4097/1
  Block4097/1 -> SubSystem1512/2(Block4101)
  Block4097/2 -> SubSystem1516/2(Block4105)
  SubSystem1512/1(Block4103) -> Block4098/1
  SubSystem1516/1(Block4107) -> Block4098/2
  Block4099/1 -> Block4108/1
  SubSystem1511/1(Output) -> Block4099/1
  Block4098/1 -> Block4099/2
  Block4095/1 -> SubSystem1516/1(Block4104), SubSystem1512/1(Block4100), SubSystem1511/1(element)

== system lib_all/SubSystem1487/SubSystem1496/SubSystem1520 ==
  Block4109 : Inport
  Block4110 : Inport  Port=2
  Block4111 : EnablePort
  Block4112 : Demux  Outputs=2
  Block4113 : Mux  Inputs=2
  Block4114 : Sum  OutDataTypeStr=double  RndMeth=Zero  SaturateOnIntegerOverflow=on  InputSameDT=on
  SubSystem1521 : Subsystem  TreatAsAtomicUnit=on  mask(***ATC.DSRVEC***){bd_version=1.01.A, r0=DSM5, r1=-1, r2=, r3=, r4=, r5=, r6=, r7=, r8=, r9=, c0=, c1=, c2=, c3=, c4=, c5=, c6=, c7=, c8=, c9=}
      mask prompts: bd_version "Version"
      mask help: これはAutoCからのブロックです．編集禁止．
      settings: OpenFcn=warndlg('変更禁止!');
      mask Help: web(ui_blockhelpmng(gcb));
      mask Display: disp('ATC.DSRVEC')
  SubSystem1522 : Subsystem
  SubSystem1526 : Subsystem
  Block4123 : Outport
  wires:
  Block4109/1 -> Block4112/1
  Block4112/1 -> SubSystem1522/2(Block4116)
  Block4112/2 -> SubSystem1526/2(Block4120)
  SubSystem1522/1(Block4118) -> Block4113/1
  SubSystem1526/1(Block4122) -> Block4113/2
  Block4114/1 -> Block4123/1
  SubSystem1521/1(Output) -> Block4114/1
  Block4113/1 -> Block4114/2
  Block4110/1 -> SubSystem1526/1(Block4119), SubSystem1522/1(Block4115), SubSystem1521/1(element)

== system lib_all/SubSystem1487/SubSystem1496/SubSystem1530 ==
  Block4124 : Inport
  Block4125 : Inport  Port=2
  Block4126 : EnablePort
  Block4127 : Demux  Outputs=2
  Block4128 : Mux  Inputs=2
  Block4129 : Sum  OutDataTypeStr=double  RndMeth=Zero  SaturateOnIntegerOverflow=on  InputSameDT=on
  SubSystem1531 : Subsystem  TreatAsAtomicUnit=on  mask(***ATC.DSRVEC***){bd_version=1.01.A, r0=DSM3, r1=-1, r2=, r3=, r4=, r5=, r6=, r7=, r8=, r9=, c0=, c1=, c2=, c3=, c4=, c5=, c6=, c7=, c8=, c9=}
      mask prompts: bd_version "Version"
      mask help: これはAutoCからのブロックです．編集禁止．
      settings: OpenFcn=warndlg('変更禁止!');
      mask Help: web(ui_blockhelpmng(gcb));
      mask Display: disp('ATC.DSRVEC')
  SubSystem1532 : Subsystem
  SubSystem1536 : Subsystem
  Block4138 : Outport
  wires:
  Block4128/1 -> Block4129/2
  SubSystem1531/1(Output) -> Block4129/1
  Block4129/1 -> Block4138/1
  SubSystem1536/1(Block4137) -> Block4128/2
  SubSystem1532/1(Block4133) -> Block4128/1
  Block4127/2 -> SubSystem1536/2(Block4135)
  Block4127/1 -> SubSystem1532/2(Block4131)
  Block4124/1 -> Block4127/1
  Block4125/1 -> SubSystem1536/1(Block4134), SubSystem1532/1(Block4130), SubSystem1531/1(element)

== system lib_all/SubSystem1540/SubSystem1543/SubSystem1544 ==
  note: ***編集禁止***
  mult : Inport
  div : Inport  Port=2
  init : Inport  Port=3
  Product : Subsystem  TreatAsAtomicUnit=on
      settings: RTWSystemCode=Inline
  Out : Outport
  wires:
  init/1 -> Product/3(init)
  div/1 -> Product/2(div)
  mult/1 -> Product/1(mult)
  Product/1(Out) -> Out/1

== system lib_all/SubSystem1540/SubSystem1543/SubSystem1545 ==
  note: ***編集禁止***
  element : Inport
  Inside_DSR : DataStoreRead  DataStoreName=DSM95
  Selector : Selector  IndexOptions=Index vector (port)  Indices=[1 3]  OutputSizes=1
  Output : Outport
  wires:
  Inside_DSR/1 -> Selector/1
  element/1 -> Selector/2
  Selector/1 -> Output/1

== system lib_all/SubSystem1540/SubSystem1543/SubSystem1546 ==
  Block4172 : Inport
  Block4173 : Inport  Port=2
  Block4174 : Inport  Port=3
  Block4175 : Sum  OutDataTypeStr=double  RndMeth=Zero  SaturateOnIntegerOverflow=on  InputSameDT=on
  Block4176 : Sum  Inputs=+-  OutDataTypeStr=double  RndMeth=Zero  SaturateOnIntegerOverflow=on  InputSameDT=on
  Block4177 : Switch  Threshold=0.5  RndMeth=Zero  SaturateOnIntegerOverflow=on  InputSameDT=on
  Block4178 : Switch  Threshold=0.5  RndMeth=Zero  SaturateOnIntegerOverflow=on  InputSameDT=on
  SubSystem1547 : Subsystem
  SubSystem1548 : Subsystem
  Block4179 : Outport
  wires:
  Block4176/1 -> Block4177/1
  Block4177/1 -> Block4179/1
  Block4175/1 -> Block4178/1
  Block4178/1 -> Block4177/3
  SubSystem1548/1(Out1) -> Block4178/2
  Block4174/1 -> SubSystem1548/2(In2)
  SubSystem1547/1(Out1) -> Block4177/2
  Block4172/1 -> SubSystem1547/2(In2), Block4176/2, Block4175/1
  Block4173/1 -> Block4175/2, Block4178/3, SubSystem1548/1(In1), Block4176/1, SubSystem1547/1(In1)

== system lib_all/SubSystem1540/SubSystem1549/SubSystem1550 ==
  note: ***編集禁止***
  In1 : Inport
  In2 : Inport  Port=2
  LO1 : Logic
  RO1 : RelationalOperator  Operator=<  InputSameDT=on
  RO2 : RelationalOperator  Operator=~=  InputSameDT=on
  single1 : DataTypeConversion  OutDataTypeStr=single  SaturateOnIntegerOverflow=on  RndMeth=Zero
  single2 : DataTypeConversion  OutDataTypeStr=single  SaturateOnIntegerOverflow=on  RndMeth=Zero
  Out1 : Outport
  wires:
  single2/1 -> RO1/2, RO2/2
  single1/1 -> RO1/1, RO2/1
  In1/1 -> single1/1
  In2/1 -> single2/1
  RO1/1 -> LO1/1
  RO2/1 -> LO1/2
  LO1/1 -> Out1/1

== system lib_all/SubSystem1540/SubSystem1549/SubSystem1551 ==
  note: ***編集禁止***
  In1 : Inport
  In2 : Inport  Port=2
  RO1 : RelationalOperator  Operator===  InputSameDT=on
  single1 : DataTypeConversion  OutDataTypeStr=single  SaturateOnIntegerOverflow=on  RndMeth=Zero
  single2 : DataTypeConversion  OutDataTypeStr=single  SaturateOnIntegerOverflow=on  RndMeth=Zero
  Out1 : Outport
  wires:
  single2/1 -> RO1/2
  single1/1 -> RO1/1
  In1/1 -> single1/1
  In2/1 -> single2/1
  RO1/1 -> Out1/1

== system lib_all/SubSystem1540/SubSystem1549/SubSystem1552 ==
  note: ***編集禁止***
  In1 : Inport
  In2 : Inport  Port=2
  RO1 : RelationalOperator  Operator===  InputSameDT=on
  single1 : DataTypeConversion  OutDataTypeStr=single  SaturateOnIntegerOverflow=on  RndMeth=Zero
  single2 : DataTypeConversion  OutDataTypeStr=single  SaturateOnIntegerOverflow=on  RndMeth=Zero
  Out1 : Outport
  wires:
  single2/1 -> RO1/2
  single1/1 -> RO1/1
  In1/1 -> single1/1
  In2/1 -> single2/1
  RO1/1 -> Out1/1

== system lib_all/SubSystem1540/SubSystem1549/SubSystem1553 ==
  note: ***編集禁止***
  In1 : Inport
  In2 : Inport  Port=2
  RO1 : RelationalOperator  Operator===  InputSameDT=on
  single1 : DataTypeConversion  OutDataTypeStr=single  SaturateOnIntegerOverflow=on  RndMeth=Zero
  single2 : DataTypeConversion  OutDataTypeStr=single  SaturateOnIntegerOverflow=on  RndMeth=Zero
  Out1 : Outport
  wires:
  single2/1 -> RO1/2
  single1/1 -> RO1/1
  In1/1 -> single1/1
  In2/1 -> single2/1
  RO1/1 -> Out1/1

== system lib_all/SubSystem1540/SubSystem1549/SubSystem1554 ==
  Block4202 : Inport
  Block4203 : Inport  Port=2
  Block4204 : EnablePort
  Block4205 : Demux  Outputs=2
  Block4206 : Mux  Inputs=2
  SubSystem1555 : Subsystem
  SubSystem1559 : Subsystem
  Block4215 : Outport
  wires:
  Block4206/1 -> Block4215/1
  SubSystem1559/1(Block4214) -> Block4206/2
  SubSystem1555/1(Block4210) -> Block4206/1
  Block4205/2 -> SubSystem1559/2(Block4212)
  Block4205/1 -> SubSystem1555/2(Block4208)
  Block4203/1 -> SubSystem1555/1(Block4207), SubSystem1559/1(Block4211)
  Block4202/1 -> Block4205/1

== system lib_all/SubSystem1540/SubSystem1549/SubSystem1563 ==
  Block4216 : Inport
  Block4217 : Inport  Port=2
  Block4218 : EnablePort
  Block4219 : Demux  Outputs=2
  Block4220 : Mux  Inputs=2
  Block4221 : Sum  OutDataTypeStr=double  RndMeth=Zero  SaturateOnIntegerOverflow=on  InputSameDT=on
  SubSystem1564 : Subsystem  TreatAsAtomicUnit=on  mask(***ATC.DSRVEC***){bd_version=1.01.A, r0=DSM1, r1=-1, r2=, r3=, r4=, r5=, r6=, r7=, r8=, r9=, c0=, c1=, c2=, c3=, c4=, c5=, c6=, c7=, c8=, c9=}
      mask prompts: bd_version "Version"
      mask help: これはAutoCからのブロックです．編集禁止．
      settings: OpenFcn=warndlg('変更禁止!');
      mask Help: web(ui_blockhelpmng(gcb));
      mask Display: disp('ATC.DSRVEC')
  SubSystem1565 : Subsystem
  SubSystem1569 : Subsystem
  Block4230 : Outport
  wires:
  Block4217/1 -> SubSystem1564/1(element), SubSystem1565/1(Block4222), SubSystem1569/1(Block4226)
  Block4220/1 -> Block4221/2
  SubSystem1564/1(Output) -> Block4221/1
  Block4221/1 -> Block4230/1
  SubSystem1569/1(Block4229) -> Block4220/2
  SubSystem1565/1(Block4225) -> Block4220/1
  Block4219/2 -> SubSystem1569/2(Block4227)
  Block4219/1 -> SubSystem1565/2(Block4223)
  Block4216/1 -> Block4219/1

== system lib_all/SubSystem1540/SubSystem1549/SubSystem1573 ==
  Block4231 : Inport
  Block4232 : Inport  Port=2
  Block4233 : EnablePort
  Block4234 : Demux  Outputs=2
  Block4235 : Mux  Inputs=2
  Block4236 : Sum  OutDataTypeStr=double  RndMeth=Zero  SaturateOnIntegerOverflow=on  InputSameDT=on
  SubSystem1574 : Subsystem  TreatAsAtomicUnit=on  mask(***ATC.DSRVEC***){bd_version=1.01.A, r0=DSM5, r1=-1, r2=, r3=, r4=, r5=, r6=, r7=, r8=, r9=, c0=, c1=, c2=, c3=, c4=, c5=, c6=, c7=, c8=, c9=}
      mask prompts: bd_version "Version"
      mask help: これはAutoCからのブロックです．編集禁止．
      settings: OpenFcn=warndlg('変更禁止!');
      mask Help: web(ui_blockhelpmng(gcb));
      mask Display: disp('ATC.DSRVEC')
  SubSystem1575 : Subsystem
  SubSystem1579 : Subsystem
  Block4245 : Outport
  wires:
  Block4232/1 -> SubSystem1574/1(element), SubSystem1575/1(Block4237), SubSystem1579/1(Block4241)
  Block4235/1 -> Block4236/2
  SubSystem1574/1(Output) -> Block4236/1
  Block4236/1 -> Block4245/1
  SubSystem1579/1(Block4244) -> Block4235/2
  SubSystem1575/1(Block4240) -> Block4235/1
  Block4234/2 -> SubSystem1579/2(Block4242)
  Block4234/1 -> SubSystem1575/2(Block4238)
  Block4231/1 -> Block4234/1

== system lib_all/SubSystem1540/SubSystem1549/SubSystem1583 ==
  Block4246 : Inport
  Block4247 : Inport  Port=2
  Block4248 : EnablePort
  Block4249 : Demux  Outputs=2
  Block4250 : Mux  Inputs=2
  Block4251 : Sum  OutDataTypeStr=double  RndMeth=Zero  SaturateOnIntegerOverflow=on  InputSameDT=on
  SubSystem1584 : Subsystem  TreatAsAtomicUnit=on  mask(***ATC.DSRVEC***){bd_version=1.01.A, r0=DSM3, r1=-1, r2=, r3=, r4=, r5=, r6=, r7=, r8=, r9=, c0=, c1=, c2=, c3=, c4=, c5=, c6=, c7=, c8=, c9=}
      mask prompts: bd_version "Version"
      mask help: これはAutoCからのブロックです．編集禁止．
      settings: OpenFcn=warndlg('変更禁止!');
      mask Help: web(ui_blockhelpmng(gcb));
      mask Display: disp('ATC.DSRVEC')
  SubSystem1585 : Subsystem
  SubSystem1589 : Subsystem
  Block4260 : Outport
  wires:
  Block4247/1 -> SubSystem1584/1(element), SubSystem1585/1(Block4252), SubSystem1589/1(Block4256)
  Block4246/1 -> Block4249/1
  Block4249/1 -> SubSystem1585/2(Block4253)
  Block4249/2 -> SubSystem1589/2(Block4257)
  SubSystem1585/1(Block4255) -> Block4250/1
  SubSystem1589/1(Block4259) -> Block4250/2
  Block4251/1 -> Block4260/1
  SubSystem1584/1(Output) -> Block4251/1
  Block4250/1 -> Block4251/2
